simulink model "vegas01_4tap_1024ch_220_r8b"  (Simulink 7.5)
settings: Solver=ode45  StartTime=0.0  StopTime=128000  RelTol=1e-3
libraries: xbsIndex_r4 x12580, monroe_library x80, xbsReplacements_r4 x42, xps_library x34, casper_library_misc x27, dspsigops x16, simulink x7
(part 16 of 91)

== system vegas01_4tap_1024ch_220_r8b/vacc2/vacc_core ==
  din_1_1 : Inport
  din_2_1 : Inport  Port=2
  din_3_1 : Inport  Port=3
  din_4_1 : Inport  Port=4
  acc_en : Inport  Port=5
  acc_mem4 : Subsystem
  adder_with_enable_1 : Subsystem
  adder_with_enable_2 : Subsystem
  adder_with_enable_3 : Subsystem
  adder_with_enable_4 : Subsystem
  dout_1_1 : Outport
  dout_2_1 : Outport  Port=2
  dout_3_1 : Outport  Port=3
  dout_4_1 : Outport  Port=4
  wires:
  acc_en/1 -> adder_with_enable_4/3(en), adder_with_enable_3/3(en), adder_with_enable_2/3(en), adder_with_enable_1/3(en)
  adder_with_enable_1/1(dout) -> acc_mem4/1(din_1_1), dout_1_1/1
  adder_with_enable_2/1(dout) -> acc_mem4/2(din_2_1), dout_2_1/1
  adder_with_enable_3/1(dout) -> acc_mem4/3(din_3_1), dout_3_1/1
  adder_with_enable_4/1(dout) -> acc_mem4/4(din_4_1), dout_4_1/1
  acc_mem4/4(dout_4_1) -> adder_with_enable_4/2(din1)
  acc_mem4/3(dout_3_1) -> adder_with_enable_3/2(din1)
  acc_mem4/2(dout_2_1) -> adder_with_enable_2/2(din1)
  acc_mem4/1(dout_1_1) -> adder_with_enable_1/2(din1)
  din_4_1/1 -> adder_with_enable_4/1(din0)
  din_3_1/1 -> adder_with_enable_3/1(din0)
  din_2_1/1 -> adder_with_enable_2/1(din0)
  din_1_1/1 -> adder_with_enable_1/1(din0)

== system vegas01_4tap_1024ch_220_r8b/vacc3/vacc_core ==
  din_1_1 : Inport
  din_2_1 : Inport  Port=2
  din_3_1 : Inport  Port=3
  din_4_1 : Inport  Port=4
  acc_en : Inport  Port=5
  acc_mem4 : Subsystem
  adder_with_enable_1 : Subsystem
  adder_with_enable_2 : Subsystem
  adder_with_enable_3 : Subsystem
  adder_with_enable_4 : Subsystem
  dout_1_1 : Outport
  dout_2_1 : Outport  Port=2
  dout_3_1 : Outport  Port=3
  dout_4_1 : Outport  Port=4
  wires:
  acc_en/1 -> adder_with_enable_4/3(en), adder_with_enable_3/3(en), adder_with_enable_2/3(en), adder_with_enable_1/3(en)
  adder_with_enable_1/1(dout) -> acc_mem4/1(din_1_1), dout_1_1/1
  adder_with_enable_2/1(dout) -> acc_mem4/2(din_2_1), dout_2_1/1
  adder_with_enable_3/1(dout) -> acc_mem4/3(din_3_1), dout_3_1/1
  adder_with_enable_4/1(dout) -> acc_mem4/4(din_4_1), dout_4_1/1
  acc_mem4/4(dout_4_1) -> adder_with_enable_4/2(din1)
  acc_mem4/3(dout_3_1) -> adder_with_enable_3/2(din1)
  acc_mem4/2(dout_2_1) -> adder_with_enable_2/2(din1)
  acc_mem4/1(dout_1_1) -> adder_with_enable_1/2(din1)
  din_4_1/1 -> adder_with_enable_4/1(din0)
  din_3_1/1 -> adder_with_enable_3/1(din0)
  din_2_1/1 -> adder_with_enable_2/1(din0)
  din_1_1/1 -> adder_with_enable_1/1(din0)

== system vegas01_4tap_1024ch_220_r8b/adc083000x2/adc0_sim/overflow_detector ==
  s0 : Inport
  s1 : Inport  Port=2
  s2 : Inport  Port=3
  s3 : Inport  Port=4
  s4 : Inport  Port=5
  s5 : Inport  Port=6
  s6 : Inport  Port=7
  s7 : Inport  Port=8
  Add : Sum  Inputs=++++
  of{0..3} (4 alike) : casper_library_misc/of1  SystemSampleTime=-1  bit_num=0  n_adc_bits=8
  overflow : Outport
  wires:
  s0/1 -> of0/1
  s4/1 -> of0/2
  of0/1 -> Add/1
  s1/1 -> of1/1
  s5/1 -> of1/2
  of1/1 -> Add/2
  s2/1 -> of2/1
  s6/1 -> of2/2
  of2/1 -> Add/3
  s3/1 -> of3/1
  s7/1 -> of3/2
  of3/1 -> Add/4
  Add/1 -> overflow/1

== system vegas01_4tap_1024ch_220_r8b/adc083000x2/adc1_sim/overflow_detector ==
  s0 : Inport
  s1 : Inport  Port=2
  s2 : Inport  Port=3
  s3 : Inport  Port=4
  s4 : Inport  Port=5
  s5 : Inport  Port=6
  s6 : Inport  Port=7
  s7 : Inport  Port=8
  Add : Sum  Inputs=++++
  of{0..3} (4 alike) : casper_library_misc/of1  SystemSampleTime=-1  bit_num=0  n_adc_bits=8
  overflow : Outport
  wires:
  s0/1 -> of0/1
  s4/1 -> of0/2
  of0/1 -> Add/1
  s1/1 -> of1/1
  s5/1 -> of1/2
  of1/1 -> Add/2
  s2/1 -> of2/1
  s6/1 -> of2/2
  of2/1 -> Add/3
  s3/1 -> of3/1
  s7/1 -> of3/2
  of3/1 -> Add/4
  Add/1 -> overflow/1

== system vegas01_4tap_1024ch_220_r8b/fft_wideband_real/fft_biplex_real_4x0/bi_real_unscr_4x ==
  sync : Inport
  even : Inport  Port=2
  odd : Inport  Port=3
  Constant0 : xbsIndex_r4/Constant  const=127  gui_display_data_type=Fixed-point  arith_type=Unsigned  n_bits=8  bin_pt=0  preci_type=Single  exp_width=8  frac_width=24  explicit_period=on  period=1  equ=P=C  opselect=C  inp2=PCIN>>17  opr=+  inp1=P  carry=CIN  dbl_ovrd=off  block_type=constant
  Constant1 : xbsIndex_r4/Constant  const=255  gui_display_data_type=Fixed-point  arith_type=Unsigned  n_bits=8  bin_pt=0  preci_type=Single  exp_width=8  frac_width=24  explicit_period=on  period=1  equ=P=C  opselect=C  inp2=PCIN>>17  opr=+  inp1=P  carry=CIN  dbl_ovrd=off  block_type=constant
  Counter0 : xbsIndex_r4/Counter  cnt_type=Free Running  cnt_to=Inf  operation=Up  start_count=1  cnt_by_val=1  arith_type=Unsigned  n_bits=8  bin_pt=0  load_pin=off  rst=on  en=off  explicit_period=on  period=1  dbl_ovrd=off  use_behavioral_HDL=off  implementation=Fabric  block_type=counter
  Delay : xbsIndex_r4/Delay  rst=off  infoeditControl=Selection of Reset will increase slice count due to use of real FFs and instead of SRLs  en=off  latency=1  dbl_ovrd=off  reg_retiming=off  block_type=delay
  Mux{0..3} (4 alike) : xbsIndex_r4/Mux  inputs=2  en=off  latency=1  precision=Full  arith_type=Unsigned  n_bits=16  bin_pt=14  quantization=Truncate  overflow=Wrap  dbl_ovrd=off  block_type=mux
  Relational0 : xbsIndex_r4/Relational  mode=a=b  en=off  latency=2  dbl_ovrd=off  block_type=relational
  Relational1 : xbsIndex_r4/Relational  mode=a=b  en=off  latency=2  dbl_ovrd=off  block_type=relational
  delay_bram0 : Subsystem
  delay_bram1 : Subsystem
  delay_ms{1..9} (9 alike) : xbsIndex_r4/Delay  rst=off  infoeditControl=Selection of Reset will increase slice count due to use of real FFs and instead of SRLs  en=off  latency=1  dbl_ovrd=off  reg_retiming=off  block_type=delay
  en_even : xbsIndex_r4/Constant  const=1  gui_display_data_type=Fixed-point  arith_type=Boolean  n_bits=1  bin_pt=0  preci_type=Single  exp_width=8  frac_width=24  explicit_period=on  period=1  equ=P=C  opselect=C  inp2=PCIN>>17  opr=+  inp1=P  carry=CIN  dbl_ovrd=off  block_type=constant
  en_odd : xbsIndex_r4/Constant  const=1  gui_display_data_type=Fixed-point  arith_type=Boolean  n_bits=1  bin_pt=0  preci_type=Single  exp_width=8  frac_width=24  explicit_period=on  period=1  equ=P=C  opselect=C  inp2=PCIN>>17  opr=+  inp1=P  carry=CIN  dbl_ovrd=off  block_type=constant
  en_out : xbsIndex_r4/Constant  const=1  gui_display_data_type=Fixed-point  arith_type=Boolean  n_bits=1  bin_pt=0  preci_type=Single  exp_width=8  frac_width=24  explicit_period=on  period=1  equ=P=C  opselect=C  inp2=PCIN>>17  opr=+  inp1=P  carry=CIN  dbl_ovrd=off  block_type=constant
  hilbert_dsp48e0 : Subsystem
  hilbert_dsp48e1 : Subsystem
  mirror_spectrum : Subsystem
  reorder_even : Subsystem
      settings: AttributesFormatString=order=2
  reorder_odd : Subsystem
      settings: AttributesFormatString=order=2
  reorder_out : Subsystem
      settings: AttributesFormatString=order=2
  sync_delay_ctr : Subsystem
  sync_out : Outport
  pol1_out : Outport  Port=2
  pol2_out : Outport  Port=3
  pol3_out : Outport  Port=4
  pol4_out : Outport  Port=5
  wires:
  sync/1 -> reorder_odd/1(sync), reorder_even/1(sync)
  reorder_even/1(sync_out) -> sync_delay_ctr/1(In), Counter0/1
  Counter0/1 -> Relational1/2, Relational0/2
  Relational0/1 -> Mux3/1, Mux0/1
  reorder_even/3(dout0) -> Mux3/3, Mux2/2, Mux1/3, Mux0/2
  Delay/1 -> Mux3/2, Mux2/3, Mux1/2, Mux0/3
  Relational1/1 -> Mux2/1, Mux1/1
  sync_delay_ctr/1(Out) -> delay_ms1/1, reorder_out/1(sync)
  delay_bram0/1(dout_1_1) -> delay_ms2/1, reorder_out/3(din0)
  delay_bram1/1(dout_1_1) -> delay_ms4/1, reorder_out/4(din1)
  hilbert_dsp48e1/1(even) -> delay_ms6/1, reorder_out/5(din2)
  hilbert_dsp48e1/2(odd) -> delay_ms8/1, reorder_out/6(din3)
  mirror_spectrum/5(dout3) -> pol4_out/1
  mirror_spectrum/4(dout2) -> pol3_out/1
  mirror_spectrum/3(dout1) -> pol2_out/1
  mirror_spectrum/2(dout0) -> pol1_out/1
  mirror_spectrum/1(sync_out) -> sync_out/1
  delay_ms9/1 -> mirror_spectrum/9(reo_in3)
  delay_ms8/1 -> mirror_spectrum/8(din3)
  delay_ms7/1 -> mirror_spectrum/7(reo_in2)
  delay_ms6/1 -> mirror_spectrum/6(din2)
  delay_ms5/1 -> mirror_spectrum/5(reo_in1)
  delay_ms4/1 -> mirror_spectrum/4(din1)
  delay_ms3/1 -> mirror_spectrum/3(reo_in0)
  delay_ms2/1 -> mirror_spectrum/2(din0)
  delay_ms1/1 -> mirror_spectrum/1(sync)
  reorder_out/6(dout3) -> delay_ms9/1
  reorder_out/5(dout2) -> delay_ms7/1
  reorder_out/4(dout1) -> delay_ms5/1
  reorder_out/3(dout0) -> delay_ms3/1
  en_out/1 -> reorder_out/2(en)
  hilbert_dsp48e0/2(odd) -> delay_bram1/1(din_1_1)
  hilbert_dsp48e0/1(even) -> delay_bram0/1(din_1_1)
  Mux3/1 -> hilbert_dsp48e1/2(b)
  Mux2/1 -> hilbert_dsp48e1/1(a)
  Mux1/1 -> hilbert_dsp48e0/2(b)
  Mux0/1 -> hilbert_dsp48e0/1(a)
  reorder_odd/3(dout0) -> Delay/1
  Constant1/1 -> Relational1/1
  Constant0/1 -> Relational0/1
  odd/1 -> reorder_odd/3(din0)
  en_odd/1 -> reorder_odd/2(en)
  even/1 -> reorder_even/3(din0)
  en_even/1 -> reorder_even/2(en)

== system vegas01_4tap_1024ch_220_r8b/fft_wideband_real/fft_biplex_real_4x0/biplex_core ==
  sync : Inport
  shift : Inport  Port=2
  pol1 : Inport  Port=3
  pol2 : Inport  Port=4
  Constant : xbsIndex_r4/Constant  const=0  gui_display_data_type=Fixed-point  arith_type=Boolean  n_bits=16  bin_pt=14  preci_type=Single  exp_width=8  frac_width=24  explicit_period=off  period=1  equ=P=C  opselect=C  inp2=PCIN>>17  opr=+  inp1=P  carry=CIN  dbl_ovrd=off  block_type=constant
  Slice{1..8} (8 alike) : xbsIndex_r4/Slice  nbits=1  boolean_output=off  mode=Upper Bit Location + Width  bit1=-0  base1=MSB of Input  bit0=0  base0=LSB of Input  dbl_ovrd=off  block_type=slice
  biplex_sel_counter : xbsIndex_r4/Counter  cnt_type=Free Running  cnt_to=Inf  operation=Up  start_count=0  cnt_by_val=1  arith_type=Unsigned  n_bits=8  bin_pt=0  load_pin=off  rst=on  en=off  explicit_period=off  period=1  dbl_ovrd=off  use_behavioral_HDL=off  implementation=Fabric  use_rpm=off  block_type=counter
  fft_stage_1 : Subsystem
      settings: AttributesFormatString=|
      | [1/8]
      | delays in BRAM
      | coeffs in slices
      | Bit growth = 0
  fft_stage_2 : Subsystem
      settings: AttributesFormatString=|
      | [2/8]
      | delays in BRAM
      | coeffs in slices
      | Bit growth = 0
  fft_stage_3 : Subsystem
      settings: AttributesFormatString=|
      | [3/8]
      | delays in BRAM
      | coeffs in slices
      | Bit growth = 0
  fft_stage_4 : Subsystem
      settings: AttributesFormatString=|
      | [4/8]
      | delays in BRAM
      | coeffs in BRAM
      | Bit growth = 0
  fft_stage_5 : Subsystem
      settings: AttributesFormatString=|
      | [5/8]
      | delays in BRAM
      | coeffs in BRAM
      | Bit growth = 0
  fft_stage_6 : Subsystem
      settings: AttributesFormatString=|
      | [6/8]
      | delays in slices
      | coeffs in BRAM
      | Bit growth = 0
  fft_stage_7 : Subsystem
      settings: AttributesFormatString=|
      | [7/8]
      | delays in slices
      | coeffs in BRAM
      | Bit growth = 0
  fft_stage_8 : Subsystem
      settings: AttributesFormatString=|
      | [8/8]
      | delays in slices
      | coeffs in BRAM
      | Bit growth = 0
  stage_2_cnt : xbsIndex_r4/Counter  cnt_type=Free Running  cnt_to=Inf  operation=Up  start_count=0  cnt_by_val=1  arith_type=Unsigned  n_bits=7  bin_pt=0  load_pin=off  rst=on  en=off  explicit_period=off  period=1  dbl_ovrd=off  use_behavioral_HDL=off  implementation=Fabric  use_rpm=off  block_type=counter
  stage_3_cnt : xbsIndex_r4/Counter  cnt_type=Free Running  cnt_to=Inf  operation=Up  start_count=0  cnt_by_val=1  arith_type=Unsigned  n_bits=6  bin_pt=0  load_pin=off  rst=on  en=off  explicit_period=off  period=1  dbl_ovrd=off  use_behavioral_HDL=off  implementation=Fabric  use_rpm=off  block_type=counter
  stage_4_cnt : xbsIndex_r4/Counter  cnt_type=Free Running  cnt_to=Inf  operation=Up  start_count=0  cnt_by_val=1  arith_type=Unsigned  n_bits=5  bin_pt=0  load_pin=off  rst=on  en=off  explicit_period=off  period=1  dbl_ovrd=off  use_behavioral_HDL=off  implementation=Fabric  use_rpm=off  block_type=counter
  stage_5_cnt : xbsIndex_r4/Counter  cnt_type=Free Running  cnt_to=Inf  operation=Up  start_count=0  cnt_by_val=1  arith_type=Unsigned  n_bits=4  bin_pt=0  load_pin=off  rst=on  en=off  explicit_period=off  period=1  dbl_ovrd=off  use_behavioral_HDL=off  implementation=Fabric  use_rpm=off  block_type=counter
  stage_6_cnt : xbsIndex_r4/Counter  cnt_type=Free Running  cnt_to=Inf  operation=Up  start_count=0  cnt_by_val=1  arith_type=Unsigned  n_bits=3  bin_pt=0  load_pin=off  rst=on  en=off  explicit_period=off  period=1  dbl_ovrd=off  use_behavioral_HDL=off  implementation=Fabric  use_rpm=off  block_type=counter
  stage_7_cnt : xbsIndex_r4/Counter  cnt_type=Free Running  cnt_to=Inf  operation=Up  start_count=0  cnt_by_val=1  arith_type=Unsigned  n_bits=2  bin_pt=0  load_pin=off  rst=on  en=off  explicit_period=off  period=1  dbl_ovrd=off  use_behavioral_HDL=off  implementation=Fabric  use_rpm=off  block_type=counter
  stage_8_cnt : xbsIndex_r4/Counter  cnt_type=Free Running  cnt_to=Inf  operation=Up  start_count=0  cnt_by_val=1  arith_type=Unsigned  n_bits=1  bin_pt=0  load_pin=off  rst=on  en=off  explicit_period=off  period=1  dbl_ovrd=off  use_behavioral_HDL=off  implementation=Fabric  use_rpm=off  block_type=counter
  sync_out : Outport
  out1 : Outport  Port=2
  out2 : Outport  Port=3
  of : Outport  Port=4
  wires:
  sync/1 -> fft_stage_1/4(sync), biplex_sel_counter/1
  shift/1 -> fft_stage_8/5(shift), fft_stage_7/5(shift), fft_stage_6/5(shift), fft_stage_5/5(shift), fft_stage_4/5(shift), fft_stage_3/5(shift), fft_stage_2/5(shift), fft_stage_1/5(shift)
  fft_stage_1/4(sync_out) -> fft_stage_2/4(sync), stage_2_cnt/1
  fft_stage_2/4(sync_out) -> fft_stage_3/4(sync), stage_3_cnt/1
  fft_stage_3/4(sync_out) -> fft_stage_4/4(sync), stage_4_cnt/1
  fft_stage_4/4(sync_out) -> fft_stage_5/4(sync), stage_5_cnt/1
  fft_stage_5/4(sync_out) -> fft_stage_6/4(sync), stage_6_cnt/1
  fft_stage_6/4(sync_out) -> fft_stage_7/4(sync), stage_7_cnt/1
  fft_stage_7/4(sync_out) -> fft_stage_8/4(sync), stage_8_cnt/1
  fft_stage_8/4(sync_out) -> sync_out/1
  fft_stage_8/3(of) -> of/1
  fft_stage_8/2(out2) -> out2/1
  fft_stage_8/1(out1) -> out1/1
  Slice8/1 -> fft_stage_8/6(biplex_sel)
  fft_stage_7/3(of) -> fft_stage_8/3(of_in)
  fft_stage_7/2(out2) -> fft_stage_8/2(in2)
  fft_stage_7/1(out1) -> fft_stage_8/1(in1)
  stage_8_cnt/1 -> Slice8/1
  Slice7/1 -> fft_stage_7/6(biplex_sel)
  fft_stage_6/3(of) -> fft_stage_7/3(of_in)
  fft_stage_6/2(out2) -> fft_stage_7/2(in2)
  fft_stage_6/1(out1) -> fft_stage_7/1(in1)
  stage_7_cnt/1 -> Slice7/1
  Slice6/1 -> fft_stage_6/6(biplex_sel)
  fft_stage_5/3(of) -> fft_stage_6/3(of_in)
  fft_stage_5/2(out2) -> fft_stage_6/2(in2)
  fft_stage_5/1(out1) -> fft_stage_6/1(in1)
  stage_6_cnt/1 -> Slice6/1
  Slice5/1 -> fft_stage_5/6(biplex_sel)
  fft_stage_4/3(of) -> fft_stage_5/3(of_in)
  fft_stage_4/2(out2) -> fft_stage_5/2(in2)
  fft_stage_4/1(out1) -> fft_stage_5/1(in1)
  stage_5_cnt/1 -> Slice5/1
  Slice4/1 -> fft_stage_4/6(biplex_sel)
  fft_stage_3/3(of) -> fft_stage_4/3(of_in)
  fft_stage_3/2(out2) -> fft_stage_4/2(in2)
  fft_stage_3/1(out1) -> fft_stage_4/1(in1)
  stage_4_cnt/1 -> Slice4/1
  Slice3/1 -> fft_stage_3/6(biplex_sel)
  fft_stage_2/3(of) -> fft_stage_3/3(of_in)
  fft_stage_2/2(out2) -> fft_stage_3/2(in2)
  fft_stage_2/1(out1) -> fft_stage_3/1(in1)
  stage_3_cnt/1 -> Slice3/1
  Slice2/1 -> fft_stage_2/6(biplex_sel)
  fft_stage_1/3(of) -> fft_stage_2/3(of_in)
  fft_stage_1/2(out2) -> fft_stage_2/2(in2)
  fft_stage_1/1(out1) -> fft_stage_2/1(in1)
  stage_2_cnt/1 -> Slice2/1
  Slice1/1 -> fft_stage_1/6(biplex_sel)
  Constant/1 -> fft_stage_1/3(of_in)
  pol2/1 -> fft_stage_1/2(in2)
  pol1/1 -> fft_stage_1/1(in1)
  biplex_sel_counter/1 -> Slice1/1

== system vegas01_4tap_1024ch_220_r8b/fft_wideband_real/fft_biplex_real_4x0/ri_to_c_pol1 ==
  re : Inport
  im : Inport  Port=2
  concat : xbsIndex_r4/Concat  num_inputs=2  dbl_ovrd=off  block_type=concat
  force_im : xbsIndex_r4/Reinterpret  force_arith_type=on  arith_type=Unsigned  force_bin_pt=on  bin_pt=0  block_type=reinterpret
  force_re : xbsIndex_r4/Reinterpret  force_arith_type=on  arith_type=Unsigned  force_bin_pt=on  bin_pt=0  block_type=reinterpret
  c : Outport
  wires:
  force_re/1 -> concat/1
  re/1 -> force_re/1
  force_im/1 -> concat/2
  im/1 -> force_im/1
  concat/1 -> c/1

== system vegas01_4tap_1024ch_220_r8b/fft_wideband_real/fft_biplex_real_4x0/ri_to_c_pol2 ==
  re : Inport
  im : Inport  Port=2
  concat : xbsIndex_r4/Concat  num_inputs=2  dbl_ovrd=off  block_type=concat
  force_im : xbsIndex_r4/Reinterpret  force_arith_type=on  arith_type=Unsigned  force_bin_pt=on  bin_pt=0  block_type=reinterpret
  force_re : xbsIndex_r4/Reinterpret  force_arith_type=on  arith_type=Unsigned  force_bin_pt=on  bin_pt=0  block_type=reinterpret
  c : Outport
  wires:
  force_re/1 -> concat/1
  re/1 -> force_re/1
  force_im/1 -> concat/2
  im/1 -> force_im/1
  concat/1 -> c/1

== system vegas01_4tap_1024ch_220_r8b/fft_wideband_real/fft_biplex_real_4x1/bi_real_unscr_4x ==
  sync : Inport
  even : Inport  Port=2
  odd : Inport  Port=3
  Constant0 : xbsIndex_r4/Constant  const=127  gui_display_data_type=Fixed-point  arith_type=Unsigned  n_bits=8  bin_pt=0  preci_type=Single  exp_width=8  frac_width=24  explicit_period=on  period=1  equ=P=C  opselect=C  inp2=PCIN>>17  opr=+  inp1=P  carry=CIN  dbl_ovrd=off  block_type=constant
  Constant1 : xbsIndex_r4/Constant  const=255  gui_display_data_type=Fixed-point  arith_type=Unsigned  n_bits=8  bin_pt=0  preci_type=Single  exp_width=8  frac_width=24  explicit_period=on  period=1  equ=P=C  opselect=C  inp2=PCIN>>17  opr=+  inp1=P  carry=CIN  dbl_ovrd=off  block_type=constant
  Counter0 : xbsIndex_r4/Counter  cnt_type=Free Running  cnt_to=Inf  operation=Up  start_count=1  cnt_by_val=1  arith_type=Unsigned  n_bits=8  bin_pt=0  load_pin=off  rst=on  en=off  explicit_period=on  period=1  dbl_ovrd=off  use_behavioral_HDL=off  implementation=Fabric  block_type=counter
  Delay : xbsIndex_r4/Delay  rst=off  infoeditControl=Selection of Reset will increase slice count due to use of real FFs and instead of SRLs  en=off  latency=1  dbl_ovrd=off  reg_retiming=off  block_type=delay
  Mux{0..3} (4 alike) : xbsIndex_r4/Mux  inputs=2  en=off  latency=1  precision=Full  arith_type=Unsigned  n_bits=16  bin_pt=14  quantization=Truncate  overflow=Wrap  dbl_ovrd=off  block_type=mux
  Relational0 : xbsIndex_r4/Relational  mode=a=b  en=off  latency=2  dbl_ovrd=off  block_type=relational
  Relational1 : xbsIndex_r4/Relational  mode=a=b  en=off  latency=2  dbl_ovrd=off  block_type=relational
  delay_bram0 : Subsystem
  delay_bram1 : Subsystem
  delay_ms{1..9} (9 alike) : xbsIndex_r4/Delay  rst=off  infoeditControl=Selection of Reset will increase slice count due to use of real FFs and instead of SRLs  en=off  latency=1  dbl_ovrd=off  reg_retiming=off  block_type=delay
  en_even : xbsIndex_r4/Constant  const=1  gui_display_data_type=Fixed-point  arith_type=Boolean  n_bits=1  bin_pt=0  preci_type=Single  exp_width=8  frac_width=24  explicit_period=on  period=1  equ=P=C  opselect=C  inp2=PCIN>>17  opr=+  inp1=P  carry=CIN  dbl_ovrd=off  block_type=constant
  en_odd : xbsIndex_r4/Constant  const=1  gui_display_data_type=Fixed-point  arith_type=Boolean  n_bits=1  bin_pt=0  preci_type=Single  exp_width=8  frac_width=24  explicit_period=on  period=1  equ=P=C  opselect=C  inp2=PCIN>>17  opr=+  inp1=P  carry=CIN  dbl_ovrd=off  block_type=constant
  en_out : xbsIndex_r4/Constant  const=1  gui_display_data_type=Fixed-point  arith_type=Boolean  n_bits=1  bin_pt=0  preci_type=Single  exp_width=8  frac_width=24  explicit_period=on  period=1  equ=P=C  opselect=C  inp2=PCIN>>17  opr=+  inp1=P  carry=CIN  dbl_ovrd=off  block_type=constant
  hilbert_dsp48e0 : Subsystem
  hilbert_dsp48e1 : Subsystem
  mirror_spectrum : Subsystem
  reorder_even : Subsystem
      settings: AttributesFormatString=order=2
  reorder_odd : Subsystem
      settings: AttributesFormatString=order=2
  reorder_out : Subsystem
      settings: AttributesFormatString=order=2
  sync_delay_ctr : Subsystem
  sync_out : Outport
  pol1_out : Outport  Port=2
  pol2_out : Outport  Port=3
  pol3_out : Outport  Port=4
  pol4_out : Outport  Port=5
  wires:
  sync/1 -> reorder_odd/1(sync), reorder_even/1(sync)
  reorder_even/1(sync_out) -> sync_delay_ctr/1(In), Counter0/1
  Counter0/1 -> Relational1/2, Relational0/2
  Relational0/1 -> Mux3/1, Mux0/1
  reorder_even/3(dout0) -> Mux3/3, Mux2/2, Mux1/3, Mux0/2
  Delay/1 -> Mux3/2, Mux2/3, Mux1/2, Mux0/3
  Relational1/1 -> Mux2/1, Mux1/1
  sync_delay_ctr/1(Out) -> delay_ms1/1, reorder_out/1(sync)
  delay_bram0/1(dout_1_1) -> delay_ms2/1, reorder_out/3(din0)
  delay_bram1/1(dout_1_1) -> delay_ms4/1, reorder_out/4(din1)
  hilbert_dsp48e1/1(even) -> delay_ms6/1, reorder_out/5(din2)
  hilbert_dsp48e1/2(odd) -> delay_ms8/1, reorder_out/6(din3)
  mirror_spectrum/5(dout3) -> pol4_out/1
  mirror_spectrum/4(dout2) -> pol3_out/1
  mirror_spectrum/3(dout1) -> pol2_out/1
  mirror_spectrum/2(dout0) -> pol1_out/1
  mirror_spectrum/1(sync_out) -> sync_out/1
  delay_ms9/1 -> mirror_spectrum/9(reo_in3)
  delay_ms8/1 -> mirror_spectrum/8(din3)
  delay_ms7/1 -> mirror_spectrum/7(reo_in2)
  delay_ms6/1 -> mirror_spectrum/6(din2)
  delay_ms5/1 -> mirror_spectrum/5(reo_in1)
  delay_ms4/1 -> mirror_spectrum/4(din1)
  delay_ms3/1 -> mirror_spectrum/3(reo_in0)
  delay_ms2/1 -> mirror_spectrum/2(din0)
  delay_ms1/1 -> mirror_spectrum/1(sync)
  reorder_out/6(dout3) -> delay_ms9/1
  reorder_out/5(dout2) -> delay_ms7/1
  reorder_out/4(dout1) -> delay_ms5/1
  reorder_out/3(dout0) -> delay_ms3/1
  en_out/1 -> reorder_out/2(en)
  hilbert_dsp48e0/2(odd) -> delay_bram1/1(din_1_1)
  hilbert_dsp48e0/1(even) -> delay_bram0/1(din_1_1)
  Mux3/1 -> hilbert_dsp48e1/2(b)
  Mux2/1 -> hilbert_dsp48e1/1(a)
  Mux1/1 -> hilbert_dsp48e0/2(b)
  Mux0/1 -> hilbert_dsp48e0/1(a)
  reorder_odd/3(dout0) -> Delay/1
  Constant1/1 -> Relational1/1
  Constant0/1 -> Relational0/1
  odd/1 -> reorder_odd/3(din0)
  en_odd/1 -> reorder_odd/2(en)
  even/1 -> reorder_even/3(din0)
  en_even/1 -> reorder_even/2(en)

== system vegas01_4tap_1024ch_220_r8b/fft_wideband_real/fft_biplex_real_4x1/biplex_core ==
  sync : Inport
  shift : Inport  Port=2
  pol1 : Inport  Port=3
  pol2 : Inport  Port=4
  Constant : xbsIndex_r4/Constant  const=0  gui_display_data_type=Fixed-point  arith_type=Boolean  n_bits=16  bin_pt=14  preci_type=Single  exp_width=8  frac_width=24  explicit_period=off  period=1  equ=P=C  opselect=C  inp2=PCIN>>17  opr=+  inp1=P  carry=CIN  dbl_ovrd=off  block_type=constant
  Slice{1..8} (8 alike) : xbsIndex_r4/Slice  nbits=1  boolean_output=off  mode=Upper Bit Location + Width  bit1=-0  base1=MSB of Input  bit0=0  base0=LSB of Input  dbl_ovrd=off  block_type=slice
  biplex_sel_counter : xbsIndex_r4/Counter  cnt_type=Free Running  cnt_to=Inf  operation=Up  start_count=0  cnt_by_val=1  arith_type=Unsigned  n_bits=8  bin_pt=0  load_pin=off  rst=on  en=off  explicit_period=off  period=1  dbl_ovrd=off  use_behavioral_HDL=off  implementation=Fabric  use_rpm=off  block_type=counter
  fft_stage_1 : Subsystem
      settings: AttributesFormatString=|
      | [1/8]
      | delays in BRAM
      | coeffs in slices
      | Bit growth = 0
  fft_stage_2 : Subsystem
      settings: AttributesFormatString=|
      | [2/8]
      | delays in BRAM
      | coeffs in slices
      | Bit growth = 0
  fft_stage_3 : Subsystem
      settings: AttributesFormatString=|
      | [3/8]
      | delays in BRAM
      | coeffs in slices
      | Bit growth = 0
  fft_stage_4 : Subsystem
      settings: AttributesFormatString=|
      | [4/8]
      | delays in BRAM
      | coeffs in BRAM
      | Bit growth = 0
  fft_stage_5 : Subsystem
      settings: AttributesFormatString=|
      | [5/8]
      | delays in BRAM
      | coeffs in BRAM
      | Bit growth = 0
  fft_stage_6 : Subsystem
      settings: AttributesFormatString=|
      | [6/8]
      | delays in slices
      | coeffs in BRAM
      | Bit growth = 0
  fft_stage_7 : Subsystem
      settings: AttributesFormatString=|
      | [7/8]
      | delays in slices
      | coeffs in BRAM
      | Bit growth = 0
  fft_stage_8 : Subsystem
      settings: AttributesFormatString=|
      | [8/8]
      | delays in slices
      | coeffs in BRAM
      | Bit growth = 0
  stage_2_cnt : xbsIndex_r4/Counter  cnt_type=Free Running  cnt_to=Inf  operation=Up  start_count=0  cnt_by_val=1  arith_type=Unsigned  n_bits=7  bin_pt=0  load_pin=off  rst=on  en=off  explicit_period=off  period=1  dbl_ovrd=off  use_behavioral_HDL=off  implementation=Fabric  use_rpm=off  block_type=counter
  stage_3_cnt : xbsIndex_r4/Counter  cnt_type=Free Running  cnt_to=Inf  operation=Up  start_count=0  cnt_by_val=1  arith_type=Unsigned  n_bits=6  bin_pt=0  load_pin=off  rst=on  en=off  explicit_period=off  period=1  dbl_ovrd=off  use_behavioral_HDL=off  implementation=Fabric  use_rpm=off  block_type=counter
  stage_4_cnt : xbsIndex_r4/Counter  cnt_type=Free Running  cnt_to=Inf  operation=Up  start_count=0  cnt_by_val=1  arith_type=Unsigned  n_bits=5  bin_pt=0  load_pin=off  rst=on  en=off  explicit_period=off  period=1  dbl_ovrd=off  use_behavioral_HDL=off  implementation=Fabric  use_rpm=off  block_type=counter
  stage_5_cnt : xbsIndex_r4/Counter  cnt_type=Free Running  cnt_to=Inf  operation=Up  start_count=0  cnt_by_val=1  arith_type=Unsigned  n_bits=4  bin_pt=0  load_pin=off  rst=on  en=off  explicit_period=off  period=1  dbl_ovrd=off  use_behavioral_HDL=off  implementation=Fabric  use_rpm=off  block_type=counter
  stage_6_cnt : xbsIndex_r4/Counter  cnt_type=Free Running  cnt_to=Inf  operation=Up  start_count=0  cnt_by_val=1  arith_type=Unsigned  n_bits=3  bin_pt=0  load_pin=off  rst=on  en=off  explicit_period=off  period=1  dbl_ovrd=off  use_behavioral_HDL=off  implementation=Fabric  use_rpm=off  block_type=counter
  stage_7_cnt : xbsIndex_r4/Counter  cnt_type=Free Running  cnt_to=Inf  operation=Up  start_count=0  cnt_by_val=1  arith_type=Unsigned  n_bits=2  bin_pt=0  load_pin=off  rst=on  en=off  explicit_period=off  period=1  dbl_ovrd=off  use_behavioral_HDL=off  implementation=Fabric  use_rpm=off  block_type=counter
  stage_8_cnt : xbsIndex_r4/Counter  cnt_type=Free Running  cnt_to=Inf  operation=Up  start_count=0  cnt_by_val=1  arith_type=Unsigned  n_bits=1  bin_pt=0  load_pin=off  rst=on  en=off  explicit_period=off  period=1  dbl_ovrd=off  use_behavioral_HDL=off  implementation=Fabric  use_rpm=off  block_type=counter
  sync_out : Outport
  out1 : Outport  Port=2
  out2 : Outport  Port=3
  of : Outport  Port=4
  wires:
  sync/1 -> fft_stage_1/4(sync), biplex_sel_counter/1
  shift/1 -> fft_stage_8/5(shift), fft_stage_7/5(shift), fft_stage_6/5(shift), fft_stage_5/5(shift), fft_stage_4/5(shift), fft_stage_3/5(shift), fft_stage_2/5(shift), fft_stage_1/5(shift)
  fft_stage_1/4(sync_out) -> fft_stage_2/4(sync), stage_2_cnt/1
  fft_stage_2/4(sync_out) -> fft_stage_3/4(sync), stage_3_cnt/1
  fft_stage_3/4(sync_out) -> fft_stage_4/4(sync), stage_4_cnt/1
  fft_stage_4/4(sync_out) -> fft_stage_5/4(sync), stage_5_cnt/1
  fft_stage_5/4(sync_out) -> fft_stage_6/4(sync), stage_6_cnt/1
  fft_stage_6/4(sync_out) -> fft_stage_7/4(sync), stage_7_cnt/1
  fft_stage_7/4(sync_out) -> fft_stage_8/4(sync), stage_8_cnt/1
  fft_stage_8/4(sync_out) -> sync_out/1
  fft_stage_8/3(of) -> of/1
  fft_stage_8/2(out2) -> out2/1
  fft_stage_8/1(out1) -> out1/1
  Slice8/1 -> fft_stage_8/6(biplex_sel)
  fft_stage_7/3(of) -> fft_stage_8/3(of_in)
  fft_stage_7/2(out2) -> fft_stage_8/2(in2)
  fft_stage_7/1(out1) -> fft_stage_8/1(in1)
  stage_8_cnt/1 -> Slice8/1
  Slice7/1 -> fft_stage_7/6(biplex_sel)
  fft_stage_6/3(of) -> fft_stage_7/3(of_in)
  fft_stage_6/2(out2) -> fft_stage_7/2(in2)
  fft_stage_6/1(out1) -> fft_stage_7/1(in1)
  stage_7_cnt/1 -> Slice7/1
  Slice6/1 -> fft_stage_6/6(biplex_sel)
  fft_stage_5/3(of) -> fft_stage_6/3(of_in)
  fft_stage_5/2(out2) -> fft_stage_6/2(in2)
  fft_stage_5/1(out1) -> fft_stage_6/1(in1)
  stage_6_cnt/1 -> Slice6/1
  Slice5/1 -> fft_stage_5/6(biplex_sel)
  fft_stage_4/3(of) -> fft_stage_5/3(of_in)
  fft_stage_4/2(out2) -> fft_stage_5/2(in2)
  fft_stage_4/1(out1) -> fft_stage_5/1(in1)
  stage_5_cnt/1 -> Slice5/1
  Slice4/1 -> fft_stage_4/6(biplex_sel)
  fft_stage_3/3(of) -> fft_stage_4/3(of_in)
  fft_stage_3/2(out2) -> fft_stage_4/2(in2)
  fft_stage_3/1(out1) -> fft_stage_4/1(in1)
  stage_4_cnt/1 -> Slice4/1
  Slice3/1 -> fft_stage_3/6(biplex_sel)
  fft_stage_2/3(of) -> fft_stage_3/3(of_in)
  fft_stage_2/2(out2) -> fft_stage_3/2(in2)
  fft_stage_2/1(out1) -> fft_stage_3/1(in1)
  stage_3_cnt/1 -> Slice3/1
  Slice2/1 -> fft_stage_2/6(biplex_sel)
  fft_stage_1/3(of) -> fft_stage_2/3(of_in)
  fft_stage_1/2(out2) -> fft_stage_2/2(in2)
  fft_stage_1/1(out1) -> fft_stage_2/1(in1)
  stage_2_cnt/1 -> Slice2/1
  Slice1/1 -> fft_stage_1/6(biplex_sel)
  Constant/1 -> fft_stage_1/3(of_in)
  pol2/1 -> fft_stage_1/2(in2)
  pol1/1 -> fft_stage_1/1(in1)
  biplex_sel_counter/1 -> Slice1/1

== system vegas01_4tap_1024ch_220_r8b/fft_wideband_real/fft_biplex_real_4x1/ri_to_c_pol1 ==
  re : Inport
  im : Inport  Port=2
  concat : xbsIndex_r4/Concat  num_inputs=2  dbl_ovrd=off  block_type=concat
  force_im : xbsIndex_r4/Reinterpret  force_arith_type=on  arith_type=Unsigned  force_bin_pt=on  bin_pt=0  block_type=reinterpret
  force_re : xbsIndex_r4/Reinterpret  force_arith_type=on  arith_type=Unsigned  force_bin_pt=on  bin_pt=0  block_type=reinterpret
  c : Outport
  wires:
  force_re/1 -> concat/1
  re/1 -> force_re/1
  force_im/1 -> concat/2
  im/1 -> force_im/1
  concat/1 -> c/1

== system vegas01_4tap_1024ch_220_r8b/fft_wideband_real/fft_biplex_real_4x1/ri_to_c_pol2 ==
  re : Inport
  im : Inport  Port=2
  concat : xbsIndex_r4/Concat  num_inputs=2  dbl_ovrd=off  block_type=concat
  force_im : xbsIndex_r4/Reinterpret  force_arith_type=on  arith_type=Unsigned  force_bin_pt=on  bin_pt=0  block_type=reinterpret
  force_re : xbsIndex_r4/Reinterpret  force_arith_type=on  arith_type=Unsigned  force_bin_pt=on  bin_pt=0  block_type=reinterpret
  c : Outport
  wires:
  force_re/1 -> concat/1
  re/1 -> force_re/1
  force_im/1 -> concat/2
  im/1 -> force_im/1
  concat/1 -> c/1

== system vegas01_4tap_1024ch_220_r8b/fft_wideband_real/fft_direct/butterfly1_0 ==
  a : Inport
  b : Inport  Port=2
  sync : Inport  Port=3
  shift : Inport  Port=4
  Logical : xbsIndex_r4/Logical  logical_function=OR  inputs=4  en=off  latency=0  precision=Full  arith_type=Unsigned  n_bits=16  bin_pt=0  align_bp=on  dbl_ovrd=off  block_type=logical
  Terminator : Terminator
  arith : Subsystem
  conv_of_1 : Subsystem
      settings: AttributesFormatString=[23,19]->[18,17]
  conv_of_2 : Subsystem
      settings: AttributesFormatString=[23,19]->[18,17]
  conv_of_3 : Subsystem
      settings: AttributesFormatString=[23,19]->[18,17]
  conv_of_4 : Subsystem
      settings: AttributesFormatString=[23,19]->[18,17]
  ri_to_c01 : Subsystem
  ri_to_c23 : Subsystem
  scale_{1..4} (4 alike) : xbsIndex_r4/Scale  scale_factor=-1  dbl_ovrd=off  block_type=scale
  sync_delay : xbsIndex_r4/Delay  rst=off  infoeditControl=Selection of Reset will increase slice count due to use of real FFs and instead of SRLs  en=off  latency=1  dbl_ovrd=off  reg_retiming=on  block_type=delay
  a+bw : Outport
  a-bw : Outport  Port=2
  of : Outport  Port=3
  sync_out : Outport  Port=4
  wires:
  sync_delay/1 -> sync_out/1
  arith/5(sync_out) -> sync_delay/1
  ri_to_c23/1(c) -> a-bw/1
  conv_of_4/1(dout) -> ri_to_c23/2(im)
  conv_of_3/1(dout) -> ri_to_c23/1(re)
  ri_to_c01/1(c) -> a+bw/1
  conv_of_2/1(dout) -> ri_to_c01/2(im)
  conv_of_1/1(dout) -> ri_to_c01/1(re)
  Logical/1 -> of/1
  conv_of_4/2(of) -> Logical/4
  conv_of_3/2(of) -> Logical/3
  conv_of_2/2(of) -> Logical/2
  conv_of_1/2(of) -> Logical/1
  scale_4/1 -> conv_of_4/1(din)
  arith/4(a-bw_im) -> scale_4/1
  scale_3/1 -> conv_of_3/1(din)
  arith/3(a-bw_re) -> scale_3/1
  scale_2/1 -> conv_of_2/1(din)
  arith/2(a+bw_im) -> scale_2/1
  scale_1/1 -> conv_of_1/1(din)
  arith/1(a+bw_re) -> scale_1/1
  shift/1 -> Terminator/1
  sync/1 -> arith/3(sync)
  b/1 -> arith/2(b)
  a/1 -> arith/1(a)

== system vegas01_4tap_1024ch_220_r8b/fft_wideband_real/fft_direct/butterfly1_1 ==
  a : Inport
  b : Inport  Port=2
  sync : Inport  Port=3
  shift : Inport  Port=4
  Logical : xbsIndex_r4/Logical  logical_function=OR  inputs=4  en=off  latency=0  precision=Full  arith_type=Unsigned  n_bits=16  bin_pt=0  align_bp=on  dbl_ovrd=off  block_type=logical
  Terminator : Terminator
  arith : Subsystem
  conv_of_1 : Subsystem
      settings: AttributesFormatString=[23,19]->[18,17]
  conv_of_2 : Subsystem
      settings: AttributesFormatString=[23,19]->[18,17]
  conv_of_3 : Subsystem
      settings: AttributesFormatString=[23,19]->[18,17]
  conv_of_4 : Subsystem
      settings: AttributesFormatString=[23,19]->[18,17]
  ri_to_c01 : Subsystem
  ri_to_c23 : Subsystem
  scale_{1..4} (4 alike) : xbsIndex_r4/Scale  scale_factor=-1  dbl_ovrd=off  block_type=scale
  sync_delay : xbsIndex_r4/Delay  rst=off  infoeditControl=Selection of Reset will increase slice count due to use of real FFs and instead of SRLs  en=off  latency=1  dbl_ovrd=off  reg_retiming=on  block_type=delay
  a+bw : Outport
  a-bw : Outport  Port=2
  of : Outport  Port=3
  sync_out : Outport  Port=4
  wires:
  sync_delay/1 -> sync_out/1
  arith/5(sync_out) -> sync_delay/1
  ri_to_c23/1(c) -> a-bw/1
  conv_of_4/1(dout) -> ri_to_c23/2(im)
  conv_of_3/1(dout) -> ri_to_c23/1(re)
  ri_to_c01/1(c) -> a+bw/1
  conv_of_2/1(dout) -> ri_to_c01/2(im)
  conv_of_1/1(dout) -> ri_to_c01/1(re)
  Logical/1 -> of/1
  conv_of_4/2(of) -> Logical/4
  conv_of_3/2(of) -> Logical/3
  conv_of_2/2(of) -> Logical/2
  conv_of_1/2(of) -> Logical/1
  scale_4/1 -> conv_of_4/1(din)
  arith/4(a-bw_im) -> scale_4/1
  scale_3/1 -> conv_of_3/1(din)
  arith/3(a-bw_re) -> scale_3/1
  scale_2/1 -> conv_of_2/1(din)
  arith/2(a+bw_im) -> scale_2/1
  scale_1/1 -> conv_of_1/1(din)
  arith/1(a+bw_re) -> scale_1/1
  shift/1 -> Terminator/1
  sync/1 -> arith/3(sync)
  b/1 -> arith/2(b)
  a/1 -> arith/1(a)

== system vegas01_4tap_1024ch_220_r8b/fft_wideband_real/fft_direct/butterfly1_2 ==
  a : Inport
  b : Inport  Port=2
  sync : Inport  Port=3
  shift : Inport  Port=4
  Logical : xbsIndex_r4/Logical  logical_function=OR  inputs=4  en=off  latency=0  precision=Full  arith_type=Unsigned  n_bits=16  bin_pt=0  align_bp=on  dbl_ovrd=off  block_type=logical
  Terminator : Terminator
  arith : Subsystem
  conv_of_1 : Subsystem
      settings: AttributesFormatString=[23,19]->[18,17]
  conv_of_2 : Subsystem
      settings: AttributesFormatString=[23,19]->[18,17]
  conv_of_3 : Subsystem
      settings: AttributesFormatString=[23,19]->[18,17]
  conv_of_4 : Subsystem
      settings: AttributesFormatString=[23,19]->[18,17]
  ri_to_c01 : Subsystem
  ri_to_c23 : Subsystem
  scale_{1..4} (4 alike) : xbsIndex_r4/Scale  scale_factor=-1  dbl_ovrd=off  block_type=scale
  sync_delay : xbsIndex_r4/Delay  rst=off  infoeditControl=Selection of Reset will increase slice count due to use of real FFs and instead of SRLs  en=off  latency=1  dbl_ovrd=off  reg_retiming=on  block_type=delay
  a+bw : Outport
  a-bw : Outport  Port=2
  of : Outport  Port=3
  sync_out : Outport  Port=4
  wires:
  sync_delay/1 -> sync_out/1
  arith/5(sync_out) -> sync_delay/1
  ri_to_c23/1(c) -> a-bw/1
  conv_of_4/1(dout) -> ri_to_c23/2(im)
  conv_of_3/1(dout) -> ri_to_c23/1(re)
  ri_to_c01/1(c) -> a+bw/1
  conv_of_2/1(dout) -> ri_to_c01/2(im)
  conv_of_1/1(dout) -> ri_to_c01/1(re)
  Logical/1 -> of/1
  conv_of_4/2(of) -> Logical/4
  conv_of_3/2(of) -> Logical/3
  conv_of_2/2(of) -> Logical/2
  conv_of_1/2(of) -> Logical/1
  scale_4/1 -> conv_of_4/1(din)
  arith/4(a-bw_im) -> scale_4/1
  scale_3/1 -> conv_of_3/1(din)
  arith/3(a-bw_re) -> scale_3/1
  scale_2/1 -> conv_of_2/1(din)
  arith/2(a+bw_im) -> scale_2/1
  scale_1/1 -> conv_of_1/1(din)
  arith/1(a+bw_re) -> scale_1/1
  shift/1 -> Terminator/1
  sync/1 -> arith/3(sync)
  b/1 -> arith/2(b)
  a/1 -> arith/1(a)

== system vegas01_4tap_1024ch_220_r8b/fft_wideband_real/fft_direct/butterfly1_3 ==
  a : Inport
  b : Inport  Port=2
  sync : Inport  Port=3
  shift : Inport  Port=4
  Logical : xbsIndex_r4/Logical  logical_function=OR  inputs=4  en=off  latency=0  precision=Full  arith_type=Unsigned  n_bits=16  bin_pt=0  align_bp=on  dbl_ovrd=off  block_type=logical
  Terminator : Terminator
  arith : Subsystem
  conv_of_1 : Subsystem
      settings: AttributesFormatString=[23,19]->[18,17]
  conv_of_2 : Subsystem
      settings: AttributesFormatString=[23,19]->[18,17]
  conv_of_3 : Subsystem
      settings: AttributesFormatString=[23,19]->[18,17]
  conv_of_4 : Subsystem
      settings: AttributesFormatString=[23,19]->[18,17]
  ri_to_c01 : Subsystem
  ri_to_c23 : Subsystem
  scale_{1..4} (4 alike) : xbsIndex_r4/Scale  scale_factor=-1  dbl_ovrd=off  block_type=scale
  sync_delay : xbsIndex_r4/Delay  rst=off  infoeditControl=Selection of Reset will increase slice count due to use of real FFs and instead of SRLs  en=off  latency=1  dbl_ovrd=off  reg_retiming=on  block_type=delay
  a+bw : Outport
  a-bw : Outport  Port=2
  of : Outport  Port=3
  sync_out : Outport  Port=4
  wires:
  sync_delay/1 -> sync_out/1
  arith/5(sync_out) -> sync_delay/1
  ri_to_c23/1(c) -> a-bw/1
  conv_of_4/1(dout) -> ri_to_c23/2(im)
  conv_of_3/1(dout) -> ri_to_c23/1(re)
  ri_to_c01/1(c) -> a+bw/1
  conv_of_2/1(dout) -> ri_to_c01/2(im)
  conv_of_1/1(dout) -> ri_to_c01/1(re)
  Logical/1 -> of/1
  conv_of_4/2(of) -> Logical/4
  conv_of_3/2(of) -> Logical/3
  conv_of_2/2(of) -> Logical/2
  conv_of_1/2(of) -> Logical/1
  scale_4/1 -> conv_of_4/1(din)
  arith/4(a-bw_im) -> scale_4/1
  scale_3/1 -> conv_of_3/1(din)
  arith/3(a-bw_re) -> scale_3/1
  scale_2/1 -> conv_of_2/1(din)
  arith/2(a+bw_im) -> scale_2/1
  scale_1/1 -> conv_of_1/1(din)
  arith/1(a+bw_re) -> scale_1/1
  shift/1 -> Terminator/1
  sync/1 -> arith/3(sync)
  b/1 -> arith/2(b)
  a/1 -> arith/1(a)

== system vegas01_4tap_1024ch_220_r8b/fft_wideband_real/fft_direct/butterfly2_0 ==
  a : Inport
  b : Inport  Port=2
  sync : Inport  Port=3
  shift : Inport  Port=4
  Logical : xbsIndex_r4/Logical  logical_function=OR  inputs=4  en=off  latency=0  precision=Full  arith_type=Unsigned  n_bits=16  bin_pt=0  align_bp=on  dbl_ovrd=off  block_type=logical
  Terminator : Terminator
  arith : Subsystem
  conv_of_1 : Subsystem
      settings: AttributesFormatString=[23,19]->[18,17]
  conv_of_2 : Subsystem
      settings: AttributesFormatString=[23,19]->[18,17]
  conv_of_3 : Subsystem
      settings: AttributesFormatString=[23,19]->[18,17]
  conv_of_4 : Subsystem
      settings: AttributesFormatString=[23,19]->[18,17]
  ri_to_c01 : Subsystem
  ri_to_c23 : Subsystem
  scale_{1..4} (4 alike) : xbsIndex_r4/Scale  scale_factor=-1  dbl_ovrd=off  block_type=scale
  sync_delay : xbsIndex_r4/Delay  rst=off  infoeditControl=Selection of Reset will increase slice count due to use of real FFs and instead of SRLs  en=off  latency=1  dbl_ovrd=off  reg_retiming=on  block_type=delay
  a+bw : Outport
  a-bw : Outport  Port=2
  of : Outport  Port=3
  sync_out : Outport  Port=4
  wires:
  sync_delay/1 -> sync_out/1
  arith/5(sync_out) -> sync_delay/1
  ri_to_c23/1(c) -> a-bw/1
  conv_of_4/1(dout) -> ri_to_c23/2(im)
  conv_of_3/1(dout) -> ri_to_c23/1(re)
  ri_to_c01/1(c) -> a+bw/1
  conv_of_2/1(dout) -> ri_to_c01/2(im)
  conv_of_1/1(dout) -> ri_to_c01/1(re)
  Logical/1 -> of/1
  conv_of_4/2(of) -> Logical/4
  conv_of_3/2(of) -> Logical/3
  conv_of_2/2(of) -> Logical/2
  conv_of_1/2(of) -> Logical/1
  scale_4/1 -> conv_of_4/1(din)
  arith/4(a-bw_im) -> scale_4/1
  scale_3/1 -> conv_of_3/1(din)
  arith/3(a-bw_re) -> scale_3/1
  scale_2/1 -> conv_of_2/1(din)
  arith/2(a+bw_im) -> scale_2/1
  scale_1/1 -> conv_of_1/1(din)
  arith/1(a+bw_re) -> scale_1/1
  shift/1 -> Terminator/1
  sync/1 -> arith/3(sync)
  b/1 -> arith/2(b)
  a/1 -> arith/1(a)

== system vegas01_4tap_1024ch_220_r8b/fft_wideband_real/fft_direct/butterfly2_1 ==
  a : Inport
  b : Inport  Port=2
  sync : Inport  Port=3
  shift : Inport  Port=4
  Logical : xbsIndex_r4/Logical  logical_function=OR  inputs=4  en=off  latency=0  precision=Full  arith_type=Unsigned  n_bits=16  bin_pt=0  align_bp=on  dbl_ovrd=off  block_type=logical
  Terminator : Terminator
  arith : Subsystem
  conv_of_1 : Subsystem
      settings: AttributesFormatString=[23,19]->[18,17]
  conv_of_2 : Subsystem
      settings: AttributesFormatString=[23,19]->[18,17]
  conv_of_3 : Subsystem
      settings: AttributesFormatString=[23,19]->[18,17]
  conv_of_4 : Subsystem
      settings: AttributesFormatString=[23,19]->[18,17]
  ri_to_c01 : Subsystem
  ri_to_c23 : Subsystem
  scale_{1..4} (4 alike) : xbsIndex_r4/Scale  scale_factor=-1  dbl_ovrd=off  block_type=scale
  sync_delay : xbsIndex_r4/Delay  rst=off  infoeditControl=Selection of Reset will increase slice count due to use of real FFs and instead of SRLs  en=off  latency=1  dbl_ovrd=off  reg_retiming=on  block_type=delay
  a+bw : Outport
  a-bw : Outport  Port=2
  of : Outport  Port=3
  sync_out : Outport  Port=4
  wires:
  sync_delay/1 -> sync_out/1
  arith/5(sync_out) -> sync_delay/1
  ri_to_c23/1(c) -> a-bw/1
  conv_of_4/1(dout) -> ri_to_c23/2(im)
  conv_of_3/1(dout) -> ri_to_c23/1(re)
  ri_to_c01/1(c) -> a+bw/1
  conv_of_2/1(dout) -> ri_to_c01/2(im)
  conv_of_1/1(dout) -> ri_to_c01/1(re)
  Logical/1 -> of/1
  conv_of_4/2(of) -> Logical/4
  conv_of_3/2(of) -> Logical/3
  conv_of_2/2(of) -> Logical/2
  conv_of_1/2(of) -> Logical/1
  scale_4/1 -> conv_of_4/1(din)
  arith/4(a-bw_im) -> scale_4/1
  scale_3/1 -> conv_of_3/1(din)
  arith/3(a-bw_re) -> scale_3/1
  scale_2/1 -> conv_of_2/1(din)
  arith/2(a+bw_im) -> scale_2/1
  scale_1/1 -> conv_of_1/1(din)
  arith/1(a+bw_re) -> scale_1/1
  shift/1 -> Terminator/1
  sync/1 -> arith/3(sync)
  b/1 -> arith/2(b)
  a/1 -> arith/1(a)

== system vegas01_4tap_1024ch_220_r8b/fft_wideband_real/fft_direct/butterfly2_2 ==
  a : Inport
  b : Inport  Port=2
  sync : Inport  Port=3
  shift : Inport  Port=4
  Logical : xbsIndex_r4/Logical  logical_function=OR  inputs=4  en=off  latency=0  precision=Full  arith_type=Unsigned  n_bits=16  bin_pt=0  align_bp=on  dbl_ovrd=off  block_type=logical
  Terminator : Terminator
  arith : Subsystem
  conv_of_1 : Subsystem
      settings: AttributesFormatString=[23,19]->[18,17]
  conv_of_2 : Subsystem
      settings: AttributesFormatString=[23,19]->[18,17]
  conv_of_3 : Subsystem
      settings: AttributesFormatString=[23,19]->[18,17]
  conv_of_4 : Subsystem
      settings: AttributesFormatString=[23,19]->[18,17]
  ri_to_c01 : Subsystem
  ri_to_c23 : Subsystem
  scale_{1..4} (4 alike) : xbsIndex_r4/Scale  scale_factor=-1  dbl_ovrd=off  block_type=scale
  sync_delay : xbsIndex_r4/Delay  rst=off  infoeditControl=Selection of Reset will increase slice count due to use of real FFs and instead of SRLs  en=off  latency=1  dbl_ovrd=off  reg_retiming=on  block_type=delay
  a+bw : Outport
  a-bw : Outport  Port=2
  of : Outport  Port=3
  sync_out : Outport  Port=4
  wires:
  sync_delay/1 -> sync_out/1
  arith/5(sync_out) -> sync_delay/1
  ri_to_c23/1(c) -> a-bw/1
  conv_of_4/1(dout) -> ri_to_c23/2(im)
  conv_of_3/1(dout) -> ri_to_c23/1(re)
  ri_to_c01/1(c) -> a+bw/1
  conv_of_2/1(dout) -> ri_to_c01/2(im)
  conv_of_1/1(dout) -> ri_to_c01/1(re)
  Logical/1 -> of/1
  conv_of_4/2(of) -> Logical/4
  conv_of_3/2(of) -> Logical/3
  conv_of_2/2(of) -> Logical/2
  conv_of_1/2(of) -> Logical/1
  scale_4/1 -> conv_of_4/1(din)
  arith/4(a-bw_im) -> scale_4/1
  scale_3/1 -> conv_of_3/1(din)
  arith/3(a-bw_re) -> scale_3/1
  scale_2/1 -> conv_of_2/1(din)
  arith/2(a+bw_im) -> scale_2/1
  scale_1/1 -> conv_of_1/1(din)
  arith/1(a+bw_re) -> scale_1/1
  shift/1 -> Terminator/1
  sync/1 -> arith/3(sync)
  b/1 -> arith/2(b)
  a/1 -> arith/1(a)

== system vegas01_4tap_1024ch_220_r8b/fft_wideband_real/fft_direct/butterfly2_3 ==
  a : Inport
  b : Inport  Port=2
  sync : Inport  Port=3
  shift : Inport  Port=4
  Logical : xbsIndex_r4/Logical  logical_function=OR  inputs=4  en=off  latency=0  precision=Full  arith_type=Unsigned  n_bits=16  bin_pt=0  align_bp=on  dbl_ovrd=off  block_type=logical
  Terminator : Terminator
  arith : Subsystem
  conv_of_1 : Subsystem
      settings: AttributesFormatString=[23,19]->[18,17]
  conv_of_2 : Subsystem
      settings: AttributesFormatString=[23,19]->[18,17]
  conv_of_3 : Subsystem
      settings: AttributesFormatString=[23,19]->[18,17]
  conv_of_4 : Subsystem
      settings: AttributesFormatString=[23,19]->[18,17]
  ri_to_c01 : Subsystem
  ri_to_c23 : Subsystem
  scale_{1..4} (4 alike) : xbsIndex_r4/Scale  scale_factor=-1  dbl_ovrd=off  block_type=scale
  sync_delay : xbsIndex_r4/Delay  rst=off  infoeditControl=Selection of Reset will increase slice count due to use of real FFs and instead of SRLs  en=off  latency=1  dbl_ovrd=off  reg_retiming=on  block_type=delay
  a+bw : Outport
  a-bw : Outport  Port=2
  of : Outport  Port=3
  sync_out : Outport  Port=4
  wires:
  sync_delay/1 -> sync_out/1
  arith/5(sync_out) -> sync_delay/1
  ri_to_c23/1(c) -> a-bw/1
  conv_of_4/1(dout) -> ri_to_c23/2(im)
  conv_of_3/1(dout) -> ri_to_c23/1(re)
  ri_to_c01/1(c) -> a+bw/1
  conv_of_2/1(dout) -> ri_to_c01/2(im)
  conv_of_1/1(dout) -> ri_to_c01/1(re)
  Logical/1 -> of/1
  conv_of_4/2(of) -> Logical/4
  conv_of_3/2(of) -> Logical/3
  conv_of_2/2(of) -> Logical/2
  conv_of_1/2(of) -> Logical/1
  scale_4/1 -> conv_of_4/1(din)
  arith/4(a-bw_im) -> scale_4/1
  scale_3/1 -> conv_of_3/1(din)
  arith/3(a-bw_re) -> scale_3/1
  scale_2/1 -> conv_of_2/1(din)
  arith/2(a+bw_im) -> scale_2/1
  scale_1/1 -> conv_of_1/1(din)
  arith/1(a+bw_re) -> scale_1/1
  shift/1 -> Terminator/1
  sync/1 -> arith/3(sync)
  b/1 -> arith/2(b)
  a/1 -> arith/1(a)

== system vegas01_4tap_1024ch_220_r8b/fft_wideband_real/fft_direct/butterfly3_0 ==
  a : Inport
  b : Inport  Port=2
  sync : Inport  Port=3
  shift : Inport  Port=4
  Logical : xbsIndex_r4/Logical  logical_function=OR  inputs=4  en=off  latency=0  precision=Full  arith_type=Unsigned  n_bits=16  bin_pt=0  align_bp=on  dbl_ovrd=off  block_type=logical
  Terminator : Terminator
  arith : Subsystem
  conv_of_1 : Subsystem
      settings: AttributesFormatString=[23,19]->[18,17]
  conv_of_2 : Subsystem
      settings: AttributesFormatString=[23,19]->[18,17]
  conv_of_3 : Subsystem
      settings: AttributesFormatString=[23,19]->[18,17]
  conv_of_4 : Subsystem
      settings: AttributesFormatString=[23,19]->[18,17]
  ri_to_c01 : Subsystem
  ri_to_c23 : Subsystem
  scale_{1..4} (4 alike) : xbsIndex_r4/Scale  scale_factor=-1  dbl_ovrd=off  block_type=scale
  sync_delay : xbsIndex_r4/Delay  rst=off  infoeditControl=Selection of Reset will increase slice count due to use of real FFs and instead of SRLs  en=off  latency=1  dbl_ovrd=off  reg_retiming=on  block_type=delay
  a+bw : Outport
  a-bw : Outport  Port=2
  of : Outport  Port=3
  sync_out : Outport  Port=4
  wires:
  sync_delay/1 -> sync_out/1
  arith/5(sync_out) -> sync_delay/1
  ri_to_c23/1(c) -> a-bw/1
  conv_of_4/1(dout) -> ri_to_c23/2(im)
  conv_of_3/1(dout) -> ri_to_c23/1(re)
  ri_to_c01/1(c) -> a+bw/1
  conv_of_2/1(dout) -> ri_to_c01/2(im)
  conv_of_1/1(dout) -> ri_to_c01/1(re)
  Logical/1 -> of/1
  conv_of_4/2(of) -> Logical/4
  conv_of_3/2(of) -> Logical/3
  conv_of_2/2(of) -> Logical/2
  conv_of_1/2(of) -> Logical/1
  scale_4/1 -> conv_of_4/1(din)
  arith/4(a-bw_im) -> scale_4/1
  scale_3/1 -> conv_of_3/1(din)
  arith/3(a-bw_re) -> scale_3/1
  scale_2/1 -> conv_of_2/1(din)
  arith/2(a+bw_im) -> scale_2/1
  scale_1/1 -> conv_of_1/1(din)
  arith/1(a+bw_re) -> scale_1/1
  shift/1 -> Terminator/1
  sync/1 -> arith/3(sync)
  b/1 -> arith/2(b)
  a/1 -> arith/1(a)
